simulink model "dds_cwg_32k_fft_wb"  (R2018a) (continued)
== system dds_cwg_32k_fft_wb == (continued)
      mask TypeOptions arith_type: Signed | Unsigned
  snap_adc : Subsystem  Tag=casper:bitsnap  AncestorBlock=casper_library_scopes/bitfield_snapshot  mask(bitsnap){io_names=[p0_d0 p0_d1 p0_d2 p0_d3 p0_d4 p0_d5 p0_d6 p0_d7], io_widths=10, io_bps=9, io_types=1, show_format=on, snap_storage=bram, snap_dram_dimm=2, snap_dram_clock=250, snap_nsamples=10, snap_data_width=128, snap_offset=off, snap_circap=off, snap_value=off, snap_ext_arm=off, snap_ext_circ=off, snap_provide_outputs=on, snap_use_dsp48=on, snap_delay=2, extra_names=timestamp, extra_widths=32, extra_bps=0, extra_types=0}
      mask prompts: io_names "Names [msb...lsb]"; io_widths "Bitwidths [msb...lsb]"; io_bps "Binary pts [msb...lsb]"; io_types "Data types [msb...lsb], ufix=0, fix=1, bool=2"; show_format "Print format string?"; snap_storage "Storage medium"; snap_dram_dimm "DRAM dimm"; snap_dram_clock "DRAM clock rate"; snap_nsamples "Number of Samples (2^?)"; snap_data_width "Data width"; snap_offset "Start delay support"; snap_circap "Circular capture support"; snap_value "Extra value capture support"; snap_ext_arm "External arm support"; snap_ext_circ "External circular capture support"; snap_provide_outputs "Provide outputs"; snap_use_dsp48 "Use DSP48s to implement counters"; snap_delay "Pre-snapshot delay"; extra_names "Names [msb...lsb]"; extra_widths "Widths [msb...lsb]"; extra_bps "Binary pts [msb...lsb]"; extra_types "Data types [msb...lsb], ufix=0, fix=1, bool=2"
      mask help:
      | A wrapped snapshot block. Allows specification of inputs to a snapblock word so the toolflow can automatically set up the scope.
      | See documentation for snapshot for explanation of parameters.
      mask init:
      | bitsnap_init(gcb);
      settings: AttributesFormatString=|
      | snap(8):f10.9,f10.9,f10.9,f10.9,f10.9,f10.9,f10.9,f10.9 = 80 bits\n128 wide, 1024 deep
      | debugID: 20387976
      mask TypeOptions snap_storage: bram | dram
      mask Callback snap_storage: bitsnap_callback();
      mask TypeOptions snap_dram_dimm: 1 | 2 | 3 | 4
      mask TypeOptions snap_dram_clock: 200 | 250 | 266 | 300 | 333
      mask TypeOptions snap_data_width: 8 | 16 | 32 | 64 | 128
      mask Callback snap_value: bitsnap_callback();
      mask Callback snap_ext_arm: bitsnap_callback();
      mask Callback snap_ext_circ: bitsnap_callback();
      mask Callback snap_provide_outputs: bitsnap_callback();
      mask DialogControl ParameterTabVar0 Prompt: Inputs
      mask DialogControl ParameterTabVar01 Prompt: Snapshot
      mask DialogControl ParameterTabVar012 Prompt: Extra Value
  ss_fft : Subsystem  Tag=casper:bitsnap  AncestorBlock=casper_library_scopes/bitfield_snapshot  mask(bitsnap){io_names=[r0 i0 r1 i1 r2 i2 r3 i3], io_widths=[10 10 10 10 10 10 10 10], io_bps=[9 9 9 9 9 9 9 9], io_types=[1 1 1 1 1 1 1 1], show_format=on, snap_storage=bram, snap_dram_dimm=2, snap_dram_clock=250, snap_nsamples=13, snap_data_width=128, snap_offset=off, snap_circap=off, snap_value=off, snap_ext_arm=off, snap_ext_circ=off, snap_provide_outputs=on, snap_use_dsp48=on, snap_delay=2, extra_names=[c d], extra_widths=[30 2], extra_bps=[0 0], extra_types=[0 0]}
      mask prompts: io_names "Names [msb...lsb]"; io_widths "Bitwidths [msb...lsb]"; io_bps "Binary pts [msb...lsb]"; io_types "Data types [msb...lsb], ufix=0, fix=1, bool=2"; show_format "Print format string?"; snap_storage "Storage medium"; snap_dram_dimm "DRAM dimm"; snap_dram_clock "DRAM clock rate"; snap_nsamples "Number of Samples (2^?)"; snap_data_width "Data width"; snap_offset "Start delay support"; snap_circap "Circular capture support"; snap_value "Extra value capture support"; snap_ext_arm "External arm support"; snap_ext_circ "External circular capture support"; snap_provide_outputs "Provide outputs"; snap_use_dsp48 "Use DSP48s to implement counters"; snap_delay "Pre-snapshot delay"; extra_names "Names [msb...lsb]"; extra_widths "Widths [msb...lsb]"; extra_bps "Binary pts [msb...lsb]"; extra_types "Data types [msb...lsb], ufix=0, fix=1, bool=2"
      mask help:
      | A wrapped snapshot block. Allows specification of inputs to a snapblock word so the toolflow can automatically set up the scope.
      | See documentation for snapshot for explanation of parameters.
      mask init:
      | bitsnap_init(gcb);
      settings: AttributesFormatString=|
      | snap(8):f10.9,f10.9,f10.9,f10.9,f10.9,f10.9,f10.9,f10.9 = 80 bits\n128 wide, 8192 deep
      | debugID: 20376222
      mask TypeOptions snap_storage: bram | dram
      mask Callback snap_storage: bitsnap_callback();
      mask TypeOptions snap_dram_dimm: 1 | 2 | 3 | 4
      mask TypeOptions snap_dram_clock: 200 | 250 | 266 | 300 | 333
      mask TypeOptions snap_data_width: 8 | 16 | 32 | 64 | 128
      mask Callback snap_value: bitsnap_callback();
      mask Callback snap_ext_arm: bitsnap_callback();
      mask Callback snap_ext_circ: bitsnap_callback();
      mask Callback snap_provide_outputs: bitsnap_callback();
      mask DialogControl ParameterTabVar0 Prompt: Inputs
      mask DialogControl ParameterTabVar01 Prompt: Snapshot
      mask DialogControl ParameterTabVar012 Prompt: Extra Value
  ss_quant : Subsystem  Tag=casper:bitsnap  AncestorBlock=casper_library_scopes/bitfield_snapshot  mask(bitsnap){io_names=[r0 i0 r1 i1 r2 i2 r3 i3], io_widths=[8 8 8 8 8 8 8 8], io_bps=[7 7 7 7 7 7 7 7], io_types=[1 1 1 1 1 1 1 1], show_format=on, snap_storage=bram, snap_dram_dimm=2, snap_dram_clock=250, snap_nsamples=13, snap_data_width=64, snap_offset=off, snap_circap=off, snap_value=off, snap_ext_arm=off, snap_ext_circ=off, snap_provide_outputs=on, snap_use_dsp48=on, snap_delay=2, extra_names=[c d], extra_widths=[30 2], extra_bps=[0 0], extra_types=[0 0]}
      mask prompts: io_names "Names [msb...lsb]"; io_widths "Bitwidths [msb...lsb]"; io_bps "Binary pts [msb...lsb]"; io_types "Data types [msb...lsb], ufix=0, fix=1, bool=2"; show_format "Print format string?"; snap_storage "Storage medium"; snap_dram_dimm "DRAM dimm"; snap_dram_clock "DRAM clock rate"; snap_nsamples "Number of Samples (2^?)"; snap_data_width "Data width"; snap_offset "Start delay support"; snap_circap "Circular capture support"; snap_value "Extra value capture support"; snap_ext_arm "External arm support"; snap_ext_circ "External circular capture support"; snap_provide_outputs "Provide outputs"; snap_use_dsp48 "Use DSP48s to implement counters"; snap_delay "Pre-snapshot delay"; extra_names "Names [msb...lsb]"; extra_widths "Widths [msb...lsb]"; extra_bps "Binary pts [msb...lsb]"; extra_types "Data types [msb...lsb], ufix=0, fix=1, bool=2"
      mask help:
      | A wrapped snapshot block. Allows specification of inputs to a snapblock word so the toolflow can automatically set up the scope.
      | See documentation for snapshot for explanation of parameters.
      mask init:
      | bitsnap_init(gcb);
      settings: AttributesFormatString=|
      | snap(8):f8.7,f8.7,f8.7,f8.7,f8.7,f8.7,f8.7,f8.7 = 64 bits\n64 wide, 8192 deep
      | debugID: 39606767
      mask TypeOptions snap_storage: bram | dram
      mask Callback snap_storage: bitsnap_callback();
      mask TypeOptions snap_dram_dimm: 1 | 2 | 3 | 4
      mask TypeOptions snap_dram_clock: 200 | 250 | 266 | 300 | 333
      mask TypeOptions snap_data_width: 8 | 16 | 32 | 64 | 128
      mask Callback snap_value: bitsnap_callback();
      mask Callback snap_ext_arm: bitsnap_callback();
      mask Callback snap_ext_circ: bitsnap_callback();
      mask Callback snap_provide_outputs: bitsnap_callback();
      mask DialogControl ParameterTabVar0 Prompt: Inputs
      mask DialogControl ParameterTabVar01 Prompt: Snapshot
      mask DialogControl ParameterTabVar012 Prompt: Extra Value
  ss_vacc0 : Subsystem  Tag=casper:bitsnap  AncestorBlock=casper_library_scopes/bitfield_snapshot  mask(bitsnap){io_names=[data dv trig], io_widths=[32 1 1], io_bps=[16 0 0], io_types=[1 2 2], show_format=on, snap_storage=bram, snap_dram_dimm=2, snap_dram_clock=250, snap_nsamples=13, snap_data_width=64, snap_offset=off, snap_circap=off, snap_value=off, snap_ext_arm=off, snap_ext_circ=off, snap_provide_outputs=on, snap_use_dsp48=on, snap_delay=2, extra_names=[c d], extra_widths=[30 2], extra_bps=[0 0], extra_types=[0 0]}
      mask prompts: io_names "Names [msb...lsb]"; io_widths "Bitwidths [msb...lsb]"; io_bps "Binary pts [msb...lsb]"; io_types "Data types [msb...lsb], ufix=0, fix=1, bool=2"; show_format "Print format string?"; snap_storage "Storage medium"; snap_dram_dimm "DRAM dimm"; snap_dram_clock "DRAM clock rate"; snap_nsamples "Number of Samples (2^?)"; snap_data_width "Data width"; snap_offset "Start delay support"; snap_circap "Circular capture support"; snap_value "Extra value capture support"; snap_ext_arm "External arm support"; snap_ext_circ "External circular capture support"; snap_provide_outputs "Provide outputs"; snap_use_dsp48 "Use DSP48s to implement counters"; snap_delay "Pre-snapshot delay"; extra_names "Names [msb...lsb]"; extra_widths "Widths [msb...lsb]"; extra_bps "Binary pts [msb...lsb]"; extra_types "Data types [msb...lsb], ufix=0, fix=1, bool=2"
      mask help:
      | A wrapped snapshot block. Allows specification of inputs to a snapblock word so the toolflow can automatically set up the scope.
      | See documentation for snapshot for explanation of parameters.
      mask init:
      | bitsnap_init(gcb);
      settings: AttributesFormatString=|
      | snap(3):f32.16,b,b = 34 bits\n64 wide, 8192 deep
      | debugID: 41246381
      mask TypeOptions snap_storage: bram | dram
      mask Callback snap_storage: bitsnap_callback();
      mask TypeOptions snap_dram_dimm: 1 | 2 | 3 | 4
      mask TypeOptions snap_dram_clock: 200 | 250 | 266 | 300 | 333
      mask TypeOptions snap_data_width: 8 | 16 | 32 | 64 | 128
      mask Callback snap_value: bitsnap_callback();
      mask Callback snap_ext_arm: bitsnap_callback();
      mask Callback snap_ext_circ: bitsnap_callback();
      mask Callback snap_provide_outputs: bitsnap_callback();
      mask DialogControl ParameterTabVar0 Prompt: Inputs
      mask DialogControl ParameterTabVar01 Prompt: Snapshot
      mask DialogControl ParameterTabVar012 Prompt: Extra Value
  ss_vacc1 : Subsystem  Tag=casper:bitsnap  AncestorBlock=casper_library_scopes/bitfield_snapshot  mask(bitsnap){io_names=[data dv trig], io_widths=[32 1 1], io_bps=[16 0 0], io_types=[1 2 2], show_format=on, snap_storage=bram, snap_dram_dimm=2, snap_dram_clock=250, snap_nsamples=13, snap_data_width=64, snap_offset=off, snap_circap=off, snap_value=off, snap_ext_arm=off, snap_ext_circ=off, snap_provide_outputs=on, snap_use_dsp48=on, snap_delay=2, extra_names=[c d], extra_widths=[30 2], extra_bps=[0 0], extra_types=[0 0]}
      mask prompts: io_names "Names [msb...lsb]"; io_widths "Bitwidths [msb...lsb]"; io_bps "Binary pts [msb...lsb]"; io_types "Data types [msb...lsb], ufix=0, fix=1, bool=2"; show_format "Print format string?"; snap_storage "Storage medium"; snap_dram_dimm "DRAM dimm"; snap_dram_clock "DRAM clock rate"; snap_nsamples "Number of Samples (2^?)"; snap_data_width "Data width"; snap_offset "Start delay support"; snap_circap "Circular capture support"; snap_value "Extra value capture support"; snap_ext_arm "External arm support"; snap_ext_circ "External circular capture support"; snap_provide_outputs "Provide outputs"; snap_use_dsp48 "Use DSP48s to implement counters"; snap_delay "Pre-snapshot delay"; extra_names "Names [msb...lsb]"; extra_widths "Widths [msb...lsb]"; extra_bps "Binary pts [msb...lsb]"; extra_types "Data types [msb...lsb], ufix=0, fix=1, bool=2"
      mask help:
      | A wrapped snapshot block. Allows specification of inputs to a snapblock word so the toolflow can automatically set up the scope.
      | See documentation for snapshot for explanation of parameters.
      mask init:
      | bitsnap_init(gcb);
      settings: AttributesFormatString=|
      | snap(3):f32.16,b,b = 34 bits\n64 wide, 8192 deep
      | debugID: 41276172
      mask TypeOptions snap_storage: bram | dram
      mask Callback snap_storage: bitsnap_callback();
      mask TypeOptions snap_dram_dimm: 1 | 2 | 3 | 4
      mask TypeOptions snap_dram_clock: 200 | 250 | 266 | 300 | 333
      mask TypeOptions snap_data_width: 8 | 16 | 32 | 64 | 128
      mask Callback snap_value: bitsnap_callback();
      mask Callback snap_ext_arm: bitsnap_callback();
      mask Callback snap_ext_circ: bitsnap_callback();
      mask Callback snap_provide_outputs: bitsnap_callback();
      mask DialogControl ParameterTabVar0 Prompt: Inputs
      mask DialogControl ParameterTabVar01 Prompt: Snapshot
      mask DialogControl ParameterTabVar012 Prompt: Extra Value
  ss_vacc2 : Subsystem  Tag=casper:bitsnap  AncestorBlock=casper_library_scopes/bitfield_snapshot  mask(bitsnap){io_names=[data dv trig], io_widths=[32 1 1], io_bps=[16 0 0], io_types=[1 2 2], show_format=on, snap_storage=bram, snap_dram_dimm=2, snap_dram_clock=250, snap_nsamples=13, snap_data_width=64, snap_offset=off, snap_circap=off, snap_value=off, snap_ext_arm=off, snap_ext_circ=off, snap_provide_outputs=on, snap_use_dsp48=on, snap_delay=2, extra_names=[c d], extra_widths=[30 2], extra_bps=[0 0], extra_types=[0 0]}
      mask prompts: io_names "Names [msb...lsb]"; io_widths "Bitwidths [msb...lsb]"; io_bps "Binary pts [msb...lsb]"; io_types "Data types [msb...lsb], ufix=0, fix=1, bool=2"; show_format "Print format string?"; snap_storage "Storage medium"; snap_dram_dimm "DRAM dimm"; snap_dram_clock "DRAM clock rate"; snap_nsamples "Number of Samples (2^?)"; snap_data_width "Data width"; snap_offset "Start delay support"; snap_circap "Circular capture support"; snap_value "Extra value capture support"; snap_ext_arm "External arm support"; snap_ext_circ "External circular capture support"; snap_provide_outputs "Provide outputs"; snap_use_dsp48 "Use DSP48s to implement counters"; snap_delay "Pre-snapshot delay"; extra_names "Names [msb...lsb]"; extra_widths "Widths [msb...lsb]"; extra_bps "Binary pts [msb...lsb]"; extra_types "Data types [msb...lsb], ufix=0, fix=1, bool=2"
      mask help:
      | A wrapped snapshot block. Allows specification of inputs to a snapblock word so the toolflow can automatically set up the scope.
      | See documentation for snapshot for explanation of parameters.
      mask init:
      | bitsnap_init(gcb);
      settings: AttributesFormatString=|
      | snap(3):f32.16,b,b = 34 bits\n64 wide, 8192 deep
      | debugID: 41305963
      mask TypeOptions snap_storage: bram | dram
      mask Callback snap_storage: bitsnap_callback();
      mask TypeOptions snap_dram_dimm: 1 | 2 | 3 | 4
      mask TypeOptions snap_dram_clock: 200 | 250 | 266 | 300 | 333
      mask TypeOptions snap_data_width: 8 | 16 | 32 | 64 | 128
      mask Callback snap_value: bitsnap_callback();
      mask Callback snap_ext_arm: bitsnap_callback();
      mask Callback snap_ext_circ: bitsnap_callback();
      mask Callback snap_provide_outputs: bitsnap_callback();
      mask DialogControl ParameterTabVar0 Prompt: Inputs
      mask DialogControl ParameterTabVar01 Prompt: Snapshot
      mask DialogControl ParameterTabVar012 Prompt: Extra Value
  ss_vacc3 : Subsystem  Tag=casper:bitsnap  AncestorBlock=casper_library_scopes/bitfield_snapshot  mask(bitsnap){io_names=[data dv trig], io_widths=[32 1 1], io_bps=[16 0 0], io_types=[1 2 2], show_format=on, snap_storage=bram, snap_dram_dimm=2, snap_dram_clock=250, snap_nsamples=13, snap_data_width=64, snap_offset=off, snap_circap=off, snap_value=off, snap_ext_arm=off, snap_ext_circ=off, snap_provide_outputs=on, snap_use_dsp48=on, snap_delay=2, extra_names=[c d], extra_widths=[30 2], extra_bps=[0 0], extra_types=[0 0]}
      mask prompts: io_names "Names [msb...lsb]"; io_widths "Bitwidths [msb...lsb]"; io_bps "Binary pts [msb...lsb]"; io_types "Data types [msb...lsb], ufix=0, fix=1, bool=2"; show_format "Print format string?"; snap_storage "Storage medium"; snap_dram_dimm "DRAM dimm"; snap_dram_clock "DRAM clock rate"; snap_nsamples "Number of Samples (2^?)"; snap_data_width "Data width"; snap_offset "Start delay support"; snap_circap "Circular capture support"; snap_value "Extra value capture support"; snap_ext_arm "External arm support"; snap_ext_circ "External circular capture support"; snap_provide_outputs "Provide outputs"; snap_use_dsp48 "Use DSP48s to implement counters"; snap_delay "Pre-snapshot delay"; extra_names "Names [msb...lsb]"; extra_widths "Widths [msb...lsb]"; extra_bps "Binary pts [msb...lsb]"; extra_types "Data types [msb...lsb], ufix=0, fix=1, bool=2"
      mask help:
      | A wrapped snapshot block. Allows specification of inputs to a snapblock word so the toolflow can automatically set up the scope.
      | See documentation for snapshot for explanation of parameters.
      mask init:
      | bitsnap_init(gcb);
      settings: AttributesFormatString=|
      | snap(3):f32.16,b,b = 34 bits\n64 wide, 8192 deep
      | debugID: 41335754
      mask TypeOptions snap_storage: bram | dram
      mask Callback snap_storage: bitsnap_callback();
      mask TypeOptions snap_dram_dimm: 1 | 2 | 3 | 4
      mask TypeOptions snap_dram_clock: 200 | 250 | 266 | 300 | 333
      mask TypeOptions snap_data_width: 8 | 16 | 32 | 64 | 128
      mask Callback snap_value: bitsnap_callback();
      mask Callback snap_ext_arm: bitsnap_callback();
      mask Callback snap_ext_circ: bitsnap_callback();
      mask Callback snap_provide_outputs: bitsnap_callback();
      mask DialogControl ParameterTabVar0 Prompt: Inputs
      mask DialogControl ParameterTabVar01 Prompt: Snapshot
      mask DialogControl ParameterTabVar012 Prompt: Extra Value
  ss_vacc_in : Subsystem  Tag=casper:bitsnap  AncestorBlock=casper_library_scopes/bitfield_snapshot  mask(bitsnap){io_names=[d0 d1 d2 d3], io_widths=[8 8 8 8], io_bps=[7 7 7 7], io_types=[1 1 1 1], show_format=on, snap_storage=bram, snap_dram_dimm=2, snap_dram_clock=250, snap_nsamples=13, snap_data_width=32, snap_offset=off, snap_circap=off, snap_value=off, snap_ext_arm=off, snap_ext_circ=off, snap_provide_outputs=on, snap_use_dsp48=on, snap_delay=2, extra_names=[c d], extra_widths=[30 2], extra_bps=[0 0], extra_types=[0 0]}
      mask prompts: io_names "Names [msb...lsb]"; io_widths "Bitwidths [msb...lsb]"; io_bps "Binary pts [msb...lsb]"; io_types "Data types [msb...lsb], ufix=0, fix=1, bool=2"; show_format "Print format string?"; snap_storage "Storage medium"; snap_dram_dimm "DRAM dimm"; snap_dram_clock "DRAM clock rate"; snap_nsamples "Number of Samples (2^?)"; snap_data_width "Data width"; snap_offset "Start delay support"; snap_circap "Circular capture support"; snap_value "Extra value capture support"; snap_ext_arm "External arm support"; snap_ext_circ "External circular capture support"; snap_provide_outputs "Provide outputs"; snap_use_dsp48 "Use DSP48s to implement counters"; snap_delay "Pre-snapshot delay"; extra_names "Names [msb...lsb]"; extra_widths "Widths [msb...lsb]"; extra_bps "Binary pts [msb...lsb]"; extra_types "Data types [msb...lsb], ufix=0, fix=1, bool=2"
      mask help:
      | A wrapped snapshot block. Allows specification of inputs to a snapblock word so the toolflow can automatically set up the scope.
      | See documentation for snapshot for explanation of parameters.
      mask init:
      | bitsnap_init(gcb);
      settings: AttributesFormatString=|
      | snap(4):f8.7,f8.7,f8.7,f8.7 = 32 bits\n32 wide, 8192 deep
      | debugID: 100288793
      mask TypeOptions snap_storage: bram | dram
      mask Callback snap_storage: bitsnap_callback();
      mask TypeOptions snap_dram_dimm: 1 | 2 | 3 | 4
      mask TypeOptions snap_dram_clock: 200 | 250 | 266 | 300 | 333
      mask TypeOptions snap_data_width: 8 | 16 | 32 | 64 | 128
      mask Callback snap_value: bitsnap_callback();
      mask Callback snap_ext_arm: bitsnap_callback();
      mask Callback snap_ext_circ: bitsnap_callback();
      mask Callback snap_provide_outputs: bitsnap_callback();
      mask DialogControl ParameterTabVar0 Prompt: Inputs
      mask DialogControl ParameterTabVar01 Prompt: Snapshot
      mask DialogControl ParameterTabVar012 Prompt: Extra Value
  trigger filter : Subsystem
  wng0 : Subsystem  mask{seed1=hex2dec('12345678'), seed2=hex2dec('87654321'), seed3=hex2dec('45612378'), seed4=hex2dec('85274163')}
      mask prompts: seed1 "Seed 1"; seed2 "Seed 2"; seed3 "Seed 3"; seed4 "Seed 4"
  tag scope: acc_len=global, acc_scale=global, cnt_rst=global, eq=global, force_gbe_txen=global, local_time_capture=global, local_time_src=global, man_sync=global, pfb_bypass=global, snap_reord_mux_we_sel=global, snap_stop_mux_sel=global, sys_rst=global, ts_tvg_en=global
  wires:
  Constant21/1 -> gbe0/8(rx_overrun_ack)
  Constant20/1 -> gbe0/7(rx_ack)
  [gbe_rst] -> pipeline99/1(d)
  [gbe_cnt_rst] -> pipeline31/1(d)
  pipeline31/1(q) -> gbe0/9(debug_rst)
  gbe0/13(rx_dest_ip) -> pipeline115/1(d)
  gbe0/14(rx_dest_port) -> pipeline116/1(d)
  [rx_dest_ip] -> pipeline/1(d)
  pipeline/1(q) -> rx_dest_ip/1(out_reg)
  gbe0/1(led_up) -> pipeline100/1(d)
  [forty_gbe_led_up] -> pipeline20/1(d)
  gbe0/2(led_rx) -> pipeline101/1(d)
  [forty_gbe_led_rx] -> pipeline22/1(d)
  gbe0/3(led_tx) -> pipeline102/1(d)
  [forty_gbe_led_tx] -> pipeline51/1(d)
  gbe0/4(tx_afull) -> pipeline103/1(d)
  gbe0/5(tx_overflow) -> pipeline104/1(d)
  gbe0/12(rx_overrun) -> pipeline114/1(d)
  pipeline20/1(q) -> gbe_status/1(out_led_up)
  pipeline22/1(q) -> gbe_status/2(out_led_rx)
  pipeline51/1(q) -> gbe_status/3(out_led_tx)
  gbe0/8(rx_source_ip) -> pipeline110/1(d)
  gbe0/9(rx_source_port) -> pipeline111/1(d)
  gbe0/6(rx_data) -> pipeline108/1(d)
  gbe0/10(rx_end_of_frame) -> pipeline112/1(d)
  gbe0/11(rx_bad_frame) -> pipeline113/1(d)
  [s256_d] -> pipeline98/1(d)
  [s256_ip] -> pipeline97/1(d)
  [s256_port] -> pipeline96/1(d)
  Logical3/1 -> gbe0/6(tx_end_of_frame)
  [s256_v] -> Logical2/2
  [s256_eof] -> Logical3/2
  Delay4/1 -> Logical2/1, Logical3/1
  [s256_eof] -> Delay4/2
  pipeline96/1(q) -> gbe0/5(tx_dest_port)
  pipeline97/1(q) -> gbe0/4(tx_dest_ip)
  pipeline98/1(q) -> gbe0/2(tx_data)
  pipeline99/1(q) -> gbe0/1(rst)
  Logical5/1 -> Delay4/1
  pipeline100/1(q) -> [forty_gbe_led_up]
  pipeline101/1(q) -> [forty_gbe_led_rx]
  pipeline102/1(q) -> [forty_gbe_led_tx]
  pipeline103/1(q) -> [forty_gbe_tx_full]
  pipeline104/1(q) -> [forty_gbe_tx_of]
  pipeline108/1(q) -> [forty_gbe_rx_data]
  pipeline110/1(q) -> [forty_gbe_rx_src_ip]
  pipeline111/1(q) -> [forty_gbe_rx_src_port]
  pipeline112/1(q) -> [forty_gbe_rx_eof]
  pipeline113/1(q) -> [forty_gbe_rx_bad]
  pipeline114/1(q) -> [forty_gbe_rx_overrun]
  pipeline115/1(q) -> [rx_dest_ip]
  pipeline116/1(q) -> [rx_dest_port]
  Logical2/1 -> gbe0/3(tx_valid)
  gbe0/7(rx_valid) -> pipeline62/1(d)
  pipeline62/1(q) -> [forty_gbe_rx_valid]
  [gbe_txen] -> Logical5/2
  [force_gbe_txen] -> Logical7/2
  Logical7/1 -> Logical5/1
  edge_detect7/1(out) -> pipeline24/1(d)
  control/7(in_cnt_rst) -> edge_detect5/1(in)
  edge_detect5/1(out) -> pipeline18/1(d)
  control/3(in_gbe_rst) -> pipeline25/1(d)
  control/1(in_sys_rst) -> pipeline23/1(d)
  pipeline24/1(q) -> [clr_status]
  pipeline25/1(q) -> [gbe_rst]
  pipeline26/1(q) -> [gbe_txen]
  pipeline17/1(q) -> [tvg_adc0_en]
  control/5(in_tvg_adc0) -> pipeline17/1(d)
  edge_detect6/1(out) -> pipeline38/1(d)
  pipeline38/1(q) -> [gbe_cnt_rst]
  control/8(in_gbe_cnt_rst) -> edge_detect6/1(in)
  control/4(in_gbe_txen) -> pipeline26/1(d)
  control/2(in_status_clr) -> edge_detect7/1(in)
  pipeline43/1(q) -> [adc_snap_arm]
  control/9(in_adc_snap_arm) -> pipeline43/1(d)
  control/10(in_adc_snap_trig_select) -> pipeline39/1(d)
  pipeline39/1(q) -> [adc_snap_trig_source]
  pipeline59/1(q) -> [ct_tvg_en]
  control/11(in_ct_tvg_en) -> pipeline59/1(d)
  pipeline52/1(q) -> [up_dbg_rst]
  control/12(in_unpack_debug_reset) -> pipeline52/1(d)
  control/13(in_cd_bypass) -> pipeline131/1(d)
  pipeline93/1(q) -> [tvg_adc1_en]
  control/6(in_tvg_adc1) -> pipeline93/1(d)
  control/14(in_gbe_stopmux) -> pipeline27/1(d)
  pipeline27/1(q) -> [gbe_stopmux]
  control/15(in_pfb_bypass) -> pipeline66/1(d)
  pipeline66/1(q) -> [pfb_bypass]
  control/16(in_ts_tvg_en) -> pipeline148/1(d)
  pipeline148/1(q) -> [ts_tvg_en]
  control/17(in_force_gbe_txen) -> pipeline21/1(d)
  pipeline21/1(q) -> [force_gbe_txen]
  control/18(in_snap_stop_mux_sel) -> pipeline127/1(d)
  control/19(in_snap_reord_mux_we_sel) -> pipeline128/1(d)
  pipeline127/1(q) -> [snap_stop_mux_sel]
  pipeline128/1(q) -> [snap_reord_mux_we_sel]
  control/20(in_local_time_source) -> pipeline16/1(d)
  edge_detect8/1(out) -> pipeline164/1(d)
  pipeline164/1(q) -> [local_time_capture]
  pipeline16/1(q) -> [local_time_src]
  control/21(in_local_time_capture) -> edge_detect8/1(in)
  control/22(in_time_diff_check_en) -> pipeline47/1(d)
  pipeline47/1(q) -> [time_diff_check_en]
  control/23(in_auto_rst_enable) -> pipeline132/1(d)
  pipeline132/1(q) -> [auto_rst_enable]
  pipeline155/1(q) -> [pipeline_stall]
  control/24(in_pipeline_stall) -> pipeline155/1(d)
  [board_in_fault] -> Inverter/1
  Inverter/1 -> Logical7/1
  Constant18/1 -> [board_in_fault]
  Constant22/1 -> [s256_d]
  Constant23/1 -> [s256_v]
  Constant24/1 -> [s256_eof]
  Constant25/1 -> [s256_port]
  Constant26/1 -> [s256_ip]
  fft_shift/1(in_fft_shift) -> pipeline4/1(d)
  pipeline4/1(q) -> Gateway Out66/1, [fft_shift]
  Delay3/1 -> bus_expand6/1(bus_in), quant0/2(din)
  quant0/3(dvalid) -> [q_dv]
  pipeline2/1(q) -> eq0/1(addr)
  pipeline41/1(q) -> Counter4/2
  Delay52/1 -> Gateway Out70/1, quant0/3(en)
  Counter4/1 -> pipeline2/1(d)
  quant0/4(of) -> [q_of0]
  Constant15/1 -> eq0/2(data_in)
  Constant27/1 -> eq0/3(we)
  Delay51/1 -> Gateway Out69/1, quant0/1(sync_in)
  quant0/2(dout) -> [q_d64_0]
  pipeline42/1(q) -> Counter4/1
  Gateway Out24/1 -> To Workspace25/1
  bus_expand3/1(msb_out8) -> Gateway Out24/1
  Gateway Out23/1 -> To Workspace23/1
  bus_expand3/2(out7) -> Gateway Out23/1
  Gateway Out25/1 -> To Workspace24/1
  bus_expand3/3(out6) -> Gateway Out25/1
  Gateway Out26/1 -> To Workspace29/1
  bus_expand3/4(out5) -> Gateway Out26/1
  Gateway Out27/1 -> To Workspace26/1
  bus_expand3/5(out4) -> Gateway Out27/1
  Gateway Out28/1 -> To Workspace32/1
  bus_expand3/6(out3) -> Gateway Out28/1
  Gateway Out29/1 -> To Workspace27/1
  bus_expand3/7(out2) -> Gateway Out29/1
  Gateway Out32/1 -> To Workspace35/1
  bus_expand3/8(lsb_out1) -> Gateway Out32/1
  [q_dv] -> Gateway Out22/1
  Gateway Out22/1 -> To Workspace22/1
  Gateway Out21/1 -> To Workspace17/1
  [q_sync] -> Gateway Out21/1
  [q_d64_0] -> bus_expand3/1(bus_in)
  Gateway Out35/1 -> To Workspace28/1
  [q_of0] -> Gateway Out35/1
  [q_d64_0] -> bus_expand4/1(bus_in)
  bus_expand4/1(msb_out8) -> [pfb_r0]
  bus_expand4/2(out7) -> [pfb_i0]
  bus_expand4/3(out6) -> [pfb_r1]
  bus_expand4/4(out5) -> [pfb_i1]
  bus_expand4/5(out4) -> [pfb_r2]
  bus_expand4/6(out3) -> [pfb_i2]
  bus_expand4/7(out2) -> [pfb_r3]
  bus_expand4/8(lsb_out1) -> [pfb_i3]
  eq/1(in_eq) -> pipeline92/1(d)
  pipeline92/1(q) -> [eq]
  Constant38/1 -> eq/1(sim_1)
  [eq] -> eq_const_bus/1(In1)
  eq_const_bus/1(Out1) -> quant0/4(coeffs)
  [pfbin_sync] -> fft_only/2(sync_in)
  [pfbin_d0] -> fft_only/4(din1), fft_only/3(din0)
  [pfbin_dv] -> fft_only/5(en)
  [fft_shift] -> fft_only/1(shift)
  Reinterpret3/1 -> Delay120/1
  Concat8/1 -> pipeline143/1(d)
  Constant57/1 -> delay_whole1/1(sim_1)
  Constant47/1 -> delay1_tl_control0/2(sim_2)
  delay0_tl_control0/2(in_load_immediate) -> pipeline135/1(d)
  phase_rate0/1(in_delta) -> Reinterpret4/1
  Constant58/1 -> phase1/1(sim_1)
  delay0_tl_control1/1(in_load_time_lsw) -> Concat6/2
  Reinterpret5/1 -> Delay44/1
  Delay115/1 -> pipeline170/1(d)
  Delay44/1 -> Concat3/1
  Constant49/1 -> Delay13/1
  pipeline173/1(q) -> Concat14/1
  pipeline169/1(q) -> Concat22/1
  delay_frac1/1(in_initial) -> Reinterpret2/1
  pipeline176/1(q) -> Concat14/4
  Delay119/1 -> pipeline174/1(d)
  Constant44/1 -> delay0_tl_control1/1(sim_1)
  Concat14/1 -> [ld_val1]
  delay1_tl_control0/1(in_arm) -> pipeline137/1(d)
  Reinterpret6/1 -> Delay119/1
  Reinterpret4/1 -> Delay121/1
  Constant41/1 -> delta_delay1/1(sim_1)
  Constant48/1 -> delay1_tl_control1/1(sim_1)
  Constant42/1 -> delay0_tl_control0/3(sim_3)
  Concat11/1 -> pipeline169/1(d)
  Delay118/1 -> Concat11/1
  pipeline134/1(q) -> [tl_delay0_arm]
  pipeline174/1(q) -> Concat14/2
  pipeline135/1(q) -> [tl_delay0_load_immediate]
  delay1_tl_control1/1(in_load_time_lsw) -> Concat8/2
  phase1/1(in_initial) -> Reinterpret9/1
  pipeline136/1(q) -> [tl_delay0_load_time]
  delay_frac0/1(in_initial) -> Reinterpret18/1
  Delay114/1 -> Concat3/2
  delay1_tl_control0/3(in_load_time_msw) -> Concat8/1
  Constant40/1 -> phase0/1(sim_1)
  Constant76/1 -> delay_frac0/1(sim_1)
  Constant43/1 -> delay0_tl_control0/2(sim_2)
  Constant75/1 -> phase_rate0/1(sim_1)
  Concat3/1 -> pipeline173/1(d)
  delta_delay1/1(in_initial) -> Reinterpret6/1
  pipeline172/1(q) -> Concat22/4
  delay_whole1/1(in_initial) -> Reinterpret5/1
  delay0_tl_control0/1(in_arm) -> pipeline134/1(d)
  pipeline171/1(q) -> Concat22/3
  phase_rate1/1(in_delta) -> Reinterpret12/1
  delta_delay0/1(in_initial) -> Reinterpret20/1
  Reinterpret20/1 -> Delay115/1
  Delay124/1 -> pipeline175/1(d)
  Reinterpret12/1 -> Delay125/1
  pipeline170/1(q) -> Concat22/2
  Delay125/1 -> pipeline176/1(d)
  Reinterpret2/1 -> Delay114/1
  Delay120/1 -> pipeline171/1(d)
  Reinterpret19/1 -> Delay118/1
  pipeline138/1(q) -> [tl_delay1_load_immediate]
  Reinterpret18/1 -> Delay42/1
  Concat22/1 -> [ld_val0]
  Delay12/1 -> delay0_tl_control0/1(sim_1)
  phase0/1(in_initial) -> Reinterpret3/1
  pipeline143/1(q) -> [tl_delay1_load_time]
  Concat6/1 -> pipeline136/1(d)
  Delay42/1 -> Concat11/2
  pipeline137/1(q) -> [tl_delay1_arm]
  Constant56/1 -> phase_rate1/1(sim_1)
  Delay121/1 -> pipeline172/1(d)
  Constant78/1 -> delta_delay0/1(sim_1)
  delay_whole0/1(in_initial) -> Reinterpret19/1
  pipeline175/1(q) -> Concat14/3
  delay0_tl_control0/3(in_load_time_msw) -> Concat6/1
  Constant50/1 -> delay_frac1/1(sim_1)
  Delay13/1 -> delay1_tl_control0/1(sim_1)
  Reinterpret9/1 -> Delay124/1
  delay1_tl_control0/2(in_load_immediate) -> pipeline138/1(d)
  Constant45/1 -> Delay12/1
  Constant46/1 -> delay1_tl_control0/3(sim_3)
  Constant31/1 -> delay_whole0/1(sim_1)
  pipeline131/1(q) -> [cd_bypass]
  man_sync/1(in_sync) -> Delay36/1
  pipeline18/1(q) -> [cnt_rst]
  [fdfs_sync] -> Delay51/1, pipeline42/1(d)
  [fdfs_d144_0] -> Delay3/1
  [fdfs_dv] -> Delay52/1, pipeline41/1(d)
  [pfb_dv] -> [fdfs_dv]
  [pfb_sync] -> [fdfs_sync]
  [pfb_d144_0] -> [fdfs_d144_0]
  edge_detect11/1(out) -> [man_sync]
  [d80_adc0] -> [pfbin_d0]
  [sync80] -> [pfbin_sync]
  [valid80] -> [pfbin_dv]
  Gateway Out18/1 -> To Workspace21/1
  bus_expand1/1(msb_out8) -> Gateway Out12/1
  Gateway Out16/1 -> To Workspace19/1
  Gateway Out17/1 -> To Workspace16/1
  Gateway Out13/1 -> To Workspace13/1
  Gateway Out11/1 -> To Workspace12/1
  Gateway Out10/1 -> To Workspace10/1
  Gateway Out12/1 -> To Workspace14/1
  bus_expand1/8(lsb_out1) -> Gateway Out18/1
  [pfb_dv] -> Gateway Out10/1
  bus_expand1/2(out7) -> Gateway Out11/1
  bus_expand1/7(out2) -> Gateway Out17/1
  Gateway Out1/1 -> To Workspace1/1
  Gateway Out15/1 -> To Workspace15/1
  Gateway Out14/1 -> To Workspace18/1
  [pfb_sync] -> Gateway Out1/1
  [pfb_d144_0] -> bus_expand9/1(bus_in), bus_expand1/1(bus_in)
  bus_expand1/5(out4) -> Gateway Out15/1
  bus_expand1/6(out3) -> Gateway Out16/1
  bus_expand1/3(out6) -> Gateway Out13/1
  bus_expand1/4(out5) -> Gateway Out14/1
  Gateway Out59/1 -> To Workspace60/1
  Constant63/1 -> pkt_len/1(sim_1)
  Constant60/1 -> gap_sel/1(sim_1)
  Delay34/1 -> [cwg_dv], Gateway Out52/1
  edge_detect2/1(out) -> cwg/1(sync), Gateway Out30/1
  Constant52/1 -> White Gaussian Noise1/2
  Gateway Out54/1 -> To Workspace55/1
  Reinterpret7/1 -> Slice2/1
  AddSub7/1 -> Mux1/3
  Constant36/1 -> cwg_scale/1(sim_1)
  Relational3/1 -> Logical1/2
  [sys_rst] -> Register2/2
  Logical1/1 -> Gateway Out55/1, Register/2, Counter1/1
  [sys_rst] -> Logical1/1
  [sys_rst] -> cwg/4(rst)
  Gateway Out31/1 -> To Workspace31/1
  Gateway Out53/1 -> To Workspace54/1
  Delay2/1 -> Register2/1, Register2/3, Delay6/1
  pipeline11/1(q) -> Mux1/1
  Delay38/1 -> [cwg80]
  Mux1/1 -> Register/1
  pipeline1/1(q) -> Mux1/2, AddSub7/1
  cwg_scale/1(in_scale) -> Gateway Out53/1, cwg/5(scale)
  gap_sel/1(in_sel) -> pipeline11/1(d)
  Gateway Out40/1 -> To Workspace40/1
  [pkt_len] -> Relational1/2
  Register/1 -> AddSub8/1, Gateway Out56/1
  Delay6/1 -> Gateway Out31/1, cwg/3(dv)
  AddSub8/1 -> Gateway Out58/1, Relational3/2
  Slice1/1 -> AddSub7/2
  freq_cwg_input/1(in_frequency) -> cwg/2(freq), Gateway Out54/1
  pkt_len_sel/1(in_sel) -> pipeline19/1(d)
  Constant68/1 -> [osc_temp_dv]
  Constant62/1 -> pkt_len_sel/1(sim_1)
  Gateway Out30/1 -> To Workspace30/1
  Slice2/1 -> AddSub1/2
  AddSub1/1 -> Mux2/3
  Mux2/1 -> Gateway Out59/1, [pkt_len]
  Constant69/1 -> gap_offset/1(sim_1)
  White Gaussian Noise1/1 -> Reinterpret1/1
  pkt_len/1(in_len) -> pipeline9/1(d)
  Counter1/1 -> Relational3/1, Relational1/1
  cwg/3(dv_out) -> Delay34/1
  cwg/2(data_out) -> Delay38/1
  [osc_temp_dv] -> Delay2/1
  gap_offset/1(in_offset) -> pipeline1/1(d)
  cwg/1(sync_out) -> Gateway Out40/1, [cwg_sync]
  Constant70/1 -> AddSub8/2
  Gateway Out52/1 -> To Workspace53/1
  Gateway Out55/1 -> To Workspace56/1
  Gateway Out58/1 -> To Workspace59/1
  pipeline19/1(q) -> Mux2/1
  Gateway Out56/1 -> To Workspace57/1
  White Gaussian Noise1/2 -> Reinterpret7/1
  Reinterpret1/1 -> Slice1/1
  pipeline9/1(q) -> AddSub1/1, Mux2/2
  Constant51/1 -> White Gaussian Noise1/1
  bus_expand2/1(msb_out8) -> Gateway Out64/1
  bus_expand2/3(out6) -> Gateway Out65/1
  From/1 -> bus_expand2/1(bus_in)
  Gateway Out66/1 -> To Workspace68/1
  Gateway Out68/1 -> To Workspace69/1
  [pfb_of] -> Gateway Out68/1
  Gateway Out69/1 -> To Workspace70/1
  Gateway Out70/1 -> To Workspace71/1
  Gateway Out74/1 -> To Workspace74/1
  Gateway Out75/1 -> To Workspace75/1
  bus_expand6/1(msb_out8) -> Gateway Out75/1
  bus_expand6/2(out7) -> Gateway Out74/1
  Gateway Out78/1 -> To Workspace78/1
  Gateway Out79/1 -> To Workspace79/1
  bus_expand6/4(out5) -> Gateway Out78/1
  bus_expand6/3(out6) -> Gateway Out79/1
  Gateway Out81/1 -> To Workspace81/1
  Gateway Out80/1 -> To Workspace80/1
  bus_expand6/6(out3) -> Gateway Out80/1
  bus_expand6/5(out4) -> Gateway Out81/1
  Gateway Out82/1 -> To Workspace82/1
  Gateway Out83/1 -> To Workspace83/1
  bus_expand6/8(lsb_out1) -> Gateway Out82/1
  bus_expand6/7(out2) -> Gateway Out83/1
  edge_detect15/1(out) -> ss_quant/10(trig)
  ss_quant/1(arm_out) -> Terminator2/1
  [pfb_i0] -> pipeline76/1(d)
  [pfb_r0] -> pipeline28/1(d)
  [pfb_i1] -> pipeline94/1(d)
  [pfb_i2] -> pipeline105/1(d)
  [pfb_i3] -> pipeline109/1(d)
  [pfb_r3] -> pipeline107/1(d)
  [pfb_r2] -> pipeline95/1(d)
  [pfb_r1] -> pipeline89/1(d)
  [q_dv] -> pipeline117/1(d)
  pipeline28/1(q) -> ss_quant/1(in_r0)
  pipeline76/1(q) -> ss_quant/2(in_i0)
  pipeline89/1(q) -> ss_quant/3(in_r1)
  pipeline94/1(q) -> ss_quant/4(in_i1)
  pipeline95/1(q) -> ss_quant/5(in_r2)
  pipeline105/1(q) -> ss_quant/6(in_i2)
  pipeline107/1(q) -> ss_quant/7(in_r3)
  pipeline109/1(q) -> ss_quant/8(in_i3)
  pipeline117/1(q) -> ss_quant/9(we)
  [q_sync] -> pipeline118/1(d)
  pipeline118/1(q) -> edge_detect15/1(in)
  Constant83/1 -> freq_cwg_input/1(sim_1)
  bus_expand7/8(lsb_out1) -> snap_adc/8(in_p0_d7)
  bus_expand7/4(out5) -> snap_adc/4(in_p0_d3)
  bus_expand7/1(msb_out8) -> snap_adc/1(in_p0_d0)
  bus_expand7/3(out6) -> snap_adc/3(in_p0_d2)
  Delay16/1 -> bus_expand7/1(bus_in)
  [valid80] -> Delay25/1
  bus_expand7/2(out7) -> snap_adc/2(in_p0_d1)
  bus_expand7/7(out2) -> snap_adc/7(in_p0_d6)
  [d80_adc0] -> Delay16/1
  Delay25/1 -> snap_adc/9(we)
  bus_expand7/6(out3) -> snap_adc/6(in_p0_d5)
  bus_expand7/5(out4) -> snap_adc/5(in_p0_d4)
  [sync80] -> Delay35/1
  Delay35/1 -> snap_adc/10(trig)
  pipeline36/1(q) -> edge_detect13/1(in)
  [pfb_sync] -> pipeline36/1(d)
  pipeline35/1(q) -> ss_fft/9(we)
  pipeline34/1(q) -> ss_fft/8(in_i3)
  pipeline33/1(q) -> ss_fft/7(in_r3)
  pipeline12/1(q) -> ss_fft/6(in_i2)
  pipeline48/1(q) -> ss_fft/5(in_r2)
  pipeline46/1(q) -> ss_fft/4(in_i1)
  pipeline44/1(q) -> ss_fft/3(in_r1)
  pipeline40/1(q) -> ss_fft/2(in_i0)
  pipeline37/1(q) -> ss_fft/1(in_r0)
  [pfb_dv] -> pipeline35/1(d)
  bus_expand9/3(out6) -> pipeline44/1(d)
  bus_expand9/5(out4) -> pipeline48/1(d)
  bus_expand9/7(out2) -> pipeline33/1(d)
  bus_expand9/8(lsb_out1) -> pipeline34/1(d)
  bus_expand9/6(out3) -> pipeline12/1(d)
  bus_expand9/4(out5) -> pipeline46/1(d)
  bus_expand9/1(msb_out8) -> pipeline37/1(d)
  bus_expand9/2(out7) -> pipeline40/1(d)
  ss_fft/1(arm_out) -> Terminator3/1
  edge_detect13/1(out) -> ss_fft/10(trig)
  bus_expand2/2(out7) -> Gateway Out8/1
  Gateway Out8/1 -> To Workspace67/1
  Gateway Out65/1 -> To Workspace8/1
  Gateway Out9/1 -> To Workspace9/1
  bus_expand2/4(out5) -> Gateway Out9/1
  Gateway Out19/1 -> To Workspace11/1
  bus_expand2/5(out4) -> Gateway Out19/1
  Gateway Out20/1 -> To Workspace20/1
  bus_expand2/6(out3) -> Gateway Out20/1
  Gateway Out38/1 -> To Workspace38/1
  bus_expand2/7(out2) -> Gateway Out38/1
  Gateway Out39/1 -> To Workspace39/1
  bus_expand2/8(lsb_out1) -> Gateway Out39/1
  Gateway Out64/1 -> To Workspace65/1
  Register2/1 -> Delay1/1
  Logical9/1 -> edge_detect2/1(in)
  Constant6/1 -> man_sync/1(sim_1)
  Delay36/1 -> edge_detect11/1(in)
  pipeline23/1(q) -> [sys_rst]
  [man_sync] -> Logical9/2, Logical9/1
  fft_only/2(dout0) -> [pfb_d144_0]
  fft_only/3(dout1) -> [pfb_d144_1]
  fft_only/4(dvalid) -> [pfb_dv]
  fft_only/5(of) -> [pfb_of]
  fft_only/1(sync_out) -> [pfb_sync]
  Constant85/1 -> fft_shift/1(sim_1)
  pipeline10/1(q) -> [wng_scale0]
  Gateway Out73/1 -> To Workspace73/1
  Gateway Out62/1 -> To Workspace64/1
  Logical20/1 -> pipeline198/1(d)
  Gateway Out42/1 -> To Workspace44/1
  Constant79/1 -> man_rst_wng/1(sim_1)
  man_rst_wng/1(in_rst) -> Logical20/2
  wng0/1(bus_out) -> bus_expand10/1(bus_in), [wng]
  bus_expand10/5(out4) -> Gateway Out51/1
  bus_expand10/2(out7) -> Gateway Out61/1
  bus_expand10/1(msb_out8) -> Gateway Out60/1
  Gateway Out67/1 -> To Workspace66/1
  Gateway Out51/1 -> To Workspace51/1
  Gateway Out61/1 -> To Workspace63/1
  [wng_scale0] -> wng0/1(scale_16_14)
  bus_expand10/8(lsb_out1) -> Gateway Out73/1
  Gateway Out63/1 -> To Workspace62/1
  bus_expand10/3(out6) -> Gateway Out42/1
  bus_expand10/6(out3) -> Gateway Out63/1
  Gateway Out45/1 -> To Workspace50/1
  bus_expand10/4(out5) -> Gateway Out45/1
  bus_expand10/7(out2) -> Gateway Out67/1
  [sys_rst] -> Logical20/1
  Gateway Out60/1 -> To Workspace61/1
  pipeline198/1(q) -> wng0/2(rst)
  scale_wng/1(in_scale) -> Gateway Out62/1, pipeline10/1(d)
  Constant80/1 -> scale_wng/1(sim_1)
  bus_convert2/1(dout) -> [d80_adc0]
  Gateway Out118/1 -> To Workspace118/1
  bus_expand8/2(lsb_out1) -> [valid80], Gateway Out118/1
  bus_addsub/1(dout) -> bus_convert2/1(din)
  [cwg80] -> bus_addsub/1(a)
  [cwg_sync] -> Concat1/1
  [cwg_dv] -> Concat1/2
  bus_expand8/1(msb_out2) -> [sync80], Gateway Out117/1
  Gateway Out117/1 -> To Workspace117/1
  Concat1/1 -> bus_addsub/3(misci)
  bus_addsub/2(misco) -> bus_convert2/2(misci)
  [wng] -> bus_addsub/2(b)
  bus_convert2/2(misco) -> bus_expand8/1(bus_in)
  Gateway Out47/1 -> To Workspace46/1
  [acc_dv] -> pipeline88/1(d)
  Gateway Out48/1 -> To Workspace47/1
  Delay32/1 -> Delay28/1
  [acc_d1] -> Gateway Out49/1
  [acc_dv] -> pipeline72/1(d)
  pipeline14/1(q) -> ss_vacc2/3(in_trig), ss_vacc2/5(trig)
  [acc_d2] -> Gateway Out41/1
  Gateway Out99/1 -> To Workspace102/1
  pipeline61/1(q) -> real_square1/1, real_square1/2
  [pfb_r1] -> Delay7/1
  pipeline87/1(q) -> ss_vacc3/1(in_data), Gateway Out50/1
  pipeline6/1(q) -> ss_vacc_in/5(we)
  [acc_scale] -> scale2/3(scale)
  Gateway Out72/1 -> To Workspace72/1
  Delay145/1 -> Delay143/1
  [acc_dv_in] -> Delay69/1
  [acc_scale] -> scale1/3(scale)
  pipeline63/1(q) -> real_square2/2, real_square2/1
  Logical4/1 -> Gateway Out2/1, Counter5/2
  Slice/1 -> Relational4/1, Relational2/1
  [pfb_r2] -> Delay8/1
  ss_vacc_in/1(arm_out) -> Terminator4/1
  Gateway Out89/1 -> To Workspace95/1
  Delay20/1 -> Delay22/1
  real_square3/1 -> AddSub6/1
  [acc_trig] -> pipeline30/1(d)
  Delay69/1 -> ?
  pipeline74/1(q) -> ss_vacc2/1(in_data), Gateway Out43/1
  Gateway Out57/1 -> To Workspace52/1
  [new_acc] -> Gateway Out5/1
  Gateway Out97/1 -> To Workspace99/1
  pipeline79/1(q) -> imag_square3/2, imag_square3/1
  pipeline85/1(q) -> Convert3/1, Convert7/1
  Gateway Out50/1 -> To Workspace42/1
  Gateway Out101/1 -> To Workspace104/1
  Register3/1 -> Gateway Out97/1, new_acc_gap/1(out_cnt)
  Counter5/1 -> pipeline29/1(d)
  [pfb_i1] -> Delay31/1
  [new_acc] -> edge_detect10/1(in)
  [acc_d2] -> pipeline74/1(d)
  Delay10/1 -> Delay33/1
  Delay29/1 -> scale1/1(real)
  pipeline55/1(q) -> ss_vacc_in/2(in_d1)
  AddSub5/1 -> pipeline85/1(d)
  pipeline80/1(q) -> real_square3/2, real_square3/1
  acc_len/1(in_reg) -> pipeline13/1(d)
  Delay142/1 -> Delay141/1
  scale/1(real_out) -> pipeline78/1(d)
  AddSub4/1 -> pipeline84/1(d)
  pipeline8/1(q) -> ss_vacc1/1(in_data), Gateway Out87/1
  [acc_trig] -> pipeline5/1(d)
  [cnt_rst] -> Logical/2
  Slice3/1 -> Relational4/2, Relational2/2
  [acc_trig] -> pipeline14/1(d)
  new_acc_cnt/1(sim_out_cnt) -> To Workspace2/1
  Delay23/1 -> Delay21/1
  imag_square1/1 -> AddSub4/2
  Delay64/1 -> simple_bram_vacc1/2(din)
  Logical6/1 -> Gateway Out89/1, Counter2/1
  pipeline86/1(q) -> Convert4/1, Convert6/1
  pipeline71/1(q) -> [acc_scale]
  [acc_dv_in] -> pipeline6/1(d)
  [acc_dv] -> pipeline75/1(d)
  pipeline58/1(q) -> ss_vacc_in/4(in_d3)
  Delay8/1 -> Delay18/1
  Delay19/1 -> Delay9/1
  Delay66/1 -> simple_bram_vacc2/2(din)
  acc_cntrl2/1(new_acc) -> [new_acc]
  Delay62/1 -> simple_bram_vacc/2(din)
  Delay18/1 -> scale2/1(real)
  pipeline13/1(q) -> [acc_len]
  Convert2/1 -> Delay64/1, Gateway Out34/1
  Relational4/1 -> Logical4/2
  Gateway Out41/1 -> To Workspace49/1
  Gateway Out71/1 -> To Workspace58/1
  [q_sync] -> Delay14/1
  pipeline53/1(q) -> ss_vacc_in/1(in_d0)
  Delay144/1 -> acc_cntrl2/4(dv), [acc_dv_in]
  [new_acc] -> Gateway Out37/1
  ss_vacc1/1(arm_out) -> Terminator6/1
  [new_acc] -> simple_bram_vacc/1(new_acc), Delay37/1
  real_square/1 -> AddSub/1
  Delay21/1 -> scale3/2(imag)
  [acc_d0] -> Gateway Out46/1
  Gateway Out6/1 -> To Workspace6/1
  Delay31/1 -> Delay11/1
  [new_acc] -> simple_bram_vacc1/1(new_acc)
  [acc_d0] -> pipeline68/1(d)
  Register1/1 -> Gateway Out92/1, Counter2/2
  Gateway Out46/1 -> To Workspace45/1
  Delay65/1 -> ?
  pipeline83/1(q) -> Gateway Out48/1, Convert5/1, Convert10/1, Slice/1, Convert1/1
  pipeline84/1(q) -> Convert2/1, Convert8/1
  simple_bram_vacc1/1(dout) -> [acc_d1]
  [q_dv] -> Delay142/1
  Convert7/1 -> Gateway Out100/1, pipeline57/1(d)
  pipeline72/1(q) -> ss_vacc1/2(in_dv), ss_vacc1/4(we)
  Delay30/1 -> Delay26/1
  Gateway Out2/1 -> To Workspace4/1
  Delay143/1 -> pipeline202/1(d)
  [acc_sync_in] -> trigger filter/3(sync)
  pipeline30/1(q) -> ss_vacc1/3(in_trig), ss_vacc1/5(trig)
  Convert1/1 -> Slice3/1, Delay62/1, Gateway Out33/1
  [acc_d3] -> pipeline87/1(d)
  Gateway Out3/1 -> To Workspace94/1
  Gateway Out34/1 -> To Workspace34/1
  pipeline69/1(q) -> ss_vacc0/2(in_dv), ss_vacc0/4(we), Gateway Out7/1
  Convert6/1 -> Gateway Out101/1, pipeline58/1(d)
  [acc_d3] -> Gateway Out57/1
  Gateway Out5/1 -> To Workspace5/1
  Relational2/1 -> Logical4/1
  pipeline7/1(q) -> ss_vacc_in/6(trig)
  pipeline57/1(q) -> ss_vacc_in/3(in_d2)
  simple_bram_vacc/2(valid) -> [acc_dv], trigger filter/1(dvalid)
  ss_vacc0/1(arm_out) -> Terminator1/1
  [acc_sync_in] -> pipeline7/1(d)
  Gateway Out36/1 -> To Workspace36/1
  Logical/1 -> acc_cntrl2/3(rst)
  Counter3/1 -> pipeline15/1(d)
  pipeline60/1(q) -> imag_square1/2, imag_square1/1
  Convert3/1 -> Delay66/1, Gateway Out36/1
  pipeline68/1(q) -> ss_vacc0/1(in_data), Gateway Out86/1
  [pfb_i0] -> Delay32/1
  real_square2/1 -> AddSub5/1
  Constant39/1 -> acc_len/1(sim_1)
  Constant37/1 -> acc_scale/1(sim_1)
  [pfb_r0] -> Delay15/1
  [acc_trig] -> pipeline3/1(d)
  [new_acc] -> simple_bram_vacc3/1(new_acc)
  Delay26/1 -> pipeline82/1(d)
  imag_square3/1 -> AddSub6/2
  [acc_d1] -> pipeline8/1(d)
  [pfb_i3] -> Delay23/1
  pipeline3/1(q) -> ss_vacc0/3(in_trig), ss_vacc0/5(trig), Gateway Out6/1
  pipeline64/1(q) -> imag_square2/1, imag_square2/2
  [pfb_r3] -> Delay20/1
  d0_overflow/1(sim_out_cnt) -> To Workspace100/1
  simple_bram_vacc3/2(valid) -> Terminator12/1
  Delay11/1 -> scale1/2(imag)
  Delay7/1 -> Delay29/1
  [acc_dv] -> Gateway Out47/1
  [acc_dv] -> pipeline69/1(d)
  Gateway Out87/1 -> To Workspace87/1
  real_square1/1 -> AddSub4/1
  pipeline15/1(q) -> new_acc_cnt/1(out_cnt)
  imag_square2/1 -> AddSub5/2
  pipeline88/1(q) -> ss_vacc3/2(in_dv), ss_vacc3/4(we)
  Counter2/1 -> Gateway Out95/1, Register3/1
  [new_acc] -> simple_bram_vacc2/1(new_acc)
  Delay68/1 -> simple_bram_vacc3/2(din)
  new_acc_gap/1(sim_out_cnt) -> To Workspace3/1
  Delay14/1 -> Delay10/1
  pipeline81/1(q) -> Delay30/1
  edge_detect10/1(out) -> Gateway Out3/1, Register3/3, Logical6/2, Register1/1, Register1/3, Counter3/2
  [acc_sync_in] -> Counter5/1
  Gateway Out7/1 -> To Workspace7/1
  simple_bram_vacc2/1(dout) -> [acc_d2]
  pipeline202/1(q) -> Delay144/1
  simple_bram_vacc3/1(dout) -> [acc_d3]
  pipeline77/1(q) -> imag_square/1, imag_square/2
  pipeline29/1(q) -> d0_overflow/1(out_cnt)
  Delay28/1 -> scale/2(imag)
  [acc_dv_in] -> Delay63/1, Gateway Out71/1
  Convert5/1 -> Gateway Out76/1
  Gateway Out44/1 -> To Workspace43/1
  ss_vacc3/1(arm_out) -> Terminator8/1
  Convert10/1 -> Gateway Out98/1, pipeline53/1(d)
  scale3/2(imag_out) -> pipeline79/1(d)
  Delay15/1 -> Delay17/1
  pipeline82/1(q) -> Delay27/1
  Gateway Out43/1 -> To Workspace41/1
  scale/2(imag_out) -> pipeline77/1(d)
  Gateway Out100/1 -> To Workspace103/1
  AddSub/1 -> pipeline83/1(d)
  simple_bram_vacc2/2(valid) -> Terminator11/1
  scale1/2(imag_out) -> pipeline60/1(d)
  Delay17/1 -> scale/1(real)
  ss_vacc2/1(arm_out) -> Terminator7/1
  Delay33/1 -> pipeline81/1(d)
  simple_bram_vacc/1(dout) -> [acc_d0]
  Convert4/1 -> Delay68/1, Gateway Out44/1
  pipeline78/1(q) -> real_square/2, real_square/1
  Gateway Out76/1 -> To Workspace76/1
  Delay22/1 -> scale3/1(real)
  Gateway Out98/1 -> To Workspace101/1
  simple_bram_vacc1/2(valid) -> Terminator9/1
  scale1/1(real_out) -> pipeline61/1(d)
  Gateway Out37/1 -> To Workspace37/1
  acc_scale/1(in_reg) -> pipeline71/1(d)
  [acc_dv_in] -> Delay67/1
  Gateway Out86/1 -> To Workspace86/1
  [pfb_i2] -> Delay19/1
  AddSub6/1 -> pipeline86/1(d)
  pipeline75/1(q) -> ss_vacc2/2(in_dv), ss_vacc2/4(we)
  Delay141/1 -> Delay146/1
  Convert8/1 -> Gateway Out99/1, pipeline55/1(d)
  Delay67/1 -> ?
  Delay9/1 -> scale2/2(imag)
  [acc_len] -> acc_cntrl2/1(acc_len)
  scale3/1(real_out) -> pipeline80/1(d)
  pipeline201/1(q) -> Delay145/1
  Delay24/1 -> acc_cntrl2/2(sync), [acc_sync_in], Logical6/1, Register1/2, Register3/2, Counter3/1, Gateway Out72/1
  imag_square/1 -> AddSub/2
  [acc_scale] -> scale/3(scale)
  scale2/1(real_out) -> pipeline63/1(d)
  Gateway Out33/1 -> To Workspace33/1
  [acc_dv_in] -> Delay65/1
  Gateway Out92/1 -> To Workspace97/1
  pipeline5/1(q) -> ss_vacc3/3(in_trig), ss_vacc3/5(trig)
  Gateway Out95/1 -> To Workspace98/1
  scale2/2(imag_out) -> pipeline64/1(d)
  Gateway Out49/1 -> To Workspace48/1
  Delay27/1 -> Logical/1, Delay24/1
  Delay146/1 -> pipeline201/1(d)
  [acc_scale] -> scale3/3(scale)
  quant0/1(sync_out) -> [q_sync]
  [cnt_rst] -> trigger filter/4(rst)
  Delay37/1 -> trigger filter/2(new_acc)
  trigger filter/1(trig) -> [acc_trig]
